simulink model "AirDirecCtrlMgr"  (R2022b)
settings: Solver=FixedStepDiscrete  StartTime=0.0  StopTime=10.0  FixedStep=0.2  RelTol=1e-3
(part 1 of 2)
settings Model: Creator=blue.ge  LastModifiedBy=blue.ge
settings DataIOCC: SaveFormat=StructureWithTime
settings OptimizationCC: DefaultParameterBehavior=Inlined  EnhancedBackFolding=on  PassReuseOutputArgsAs=Individual arguments  ZeroExternalMemoryAtStartup=off  ZeroInternalMemoryAtStartup=off  OptimizeBlockOrder=speed  DifferentSizesBufferReuse=on  OptimizationCustomize=off  EfficientTunableParamExpr=on
settings DebuggingCC: ParameterTunabilityLossMsg=error
settings ModelReferenceCC: ModelReferenceNumInstancesAllowed=Single
settings SFSimCC: MATLABDynamicMemAlloc=off
settings RTWCC: Description=AUTOSAR  SystemTargetFile=autosar.tlc  GenCodeOnly=on  TemplateMakefile=ert_default_tmf
settings CodeAppCC: ForceParamTrailComments=on  IgnoreCustomStorageClasses=off  OperatorAnnotations=on  SimulinkDataObjDesc=on  SFDataObjDesc=on  InsertBlockDesc=on
settings STFCustomTargetCC: TargetLangStandard=C99 (ISO)  MultiwordLength=256  ParMdlRefBuildCompliant=on  CompOptLevelCompliant=on  IncludeMdlTerminateFcn=off  CombineOutputUpdateFcns=on  SuppressErrorStatus=on  ERTCustomFileBanners=on  SupportNonFinite=off  SupportComplex=off  SupportContinuousTime=off  SupportNonInlinedSFcns=off  RemoveResetFunc=on  MATLABClassNameForMDSCustomization=Simulink.SoftwareTarget.ERTCustomization  AutosarCompliant=on  UseToolchainInfoCompliant=on  LookupTableObjectStructAxisOrder=2,1,3,4,...  LUTObjectStructOrderEvenSpacing=Size,Table,Breakpoints  SystemTargetFile=autosar.tlc
settings ERTTargetCC: TargetLangStandard=C99 (ISO)  ConcurrentExecutionCompliant=off  IncludeMdlTerminateFcn=off  SuppressErrorStatus=on  SupportNonFinite=off  SupportComplex=off  ModelStepFunctionPrototypeControlCompliant=off  CPPClassGenCompliant=off  AutosarCompliant=on  LookupTableObjectStructAxisOrder=2,1,3,4,...  LUTObjectStructOrderEvenSpacing=Size,Table,Breakpoints  InlinedParameterPlacement=NonHierarchical  ERTCustomFileTemplate=example_file_process.tlc

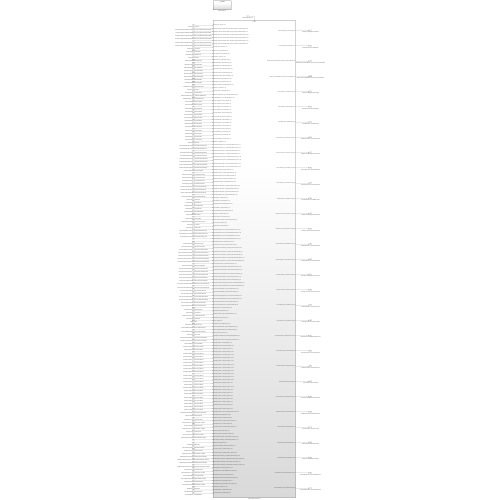
[thumbnail: Simulink's stored preview of the top-level diagram]
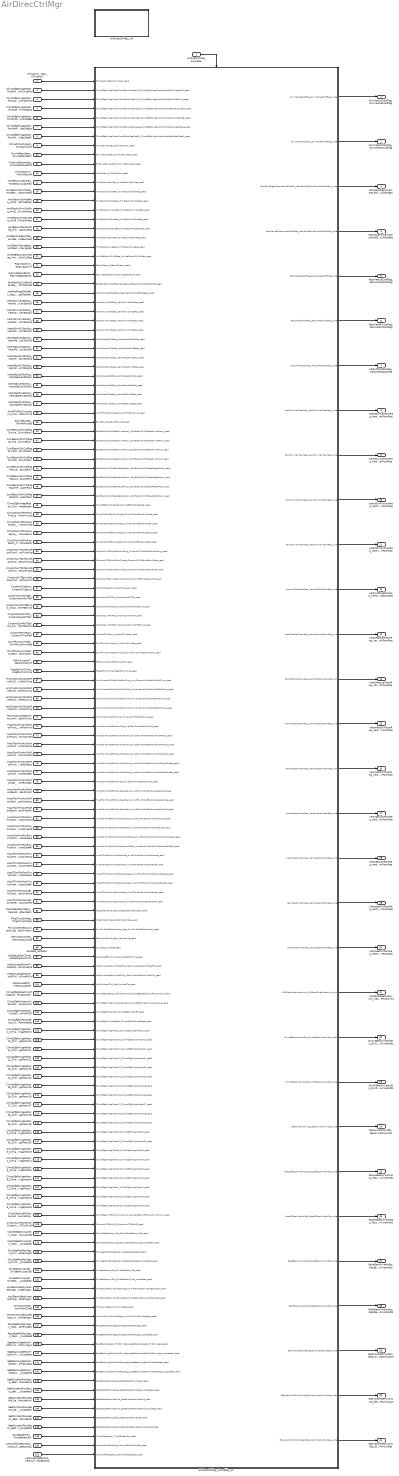
== system AirDirecCtrlMgr ==
  AirDirecCtrlMgr_Runnable : Inport  SampleTime=0.2  OutputFunctionCall=on
  ClimaActv_Ngcc_ClimaActv : Inport  Port=2  OutDataTypeStr=Enum: OnOff1  PortDimensions=1
  ClimaCfgForVgaMotorPrsntAtRowFirstLeMid_ClimaCfgForVgaMotorPrsntAtRowFirstLeMid : Inport  Port=3  OutDataTypeStr=Boolean  PortDimensions=1
  ClimaCfgForVgaMotorPrsntAtRowFirstRiMid_ClimaCfgForVgaMotorPrsntAtRowFirstRiMid : Inport  Port=4  OutDataTypeStr=Boolean  PortDimensions=1
  ClimaCfgForVgaMotorPrsntAtRowFirstSideLe_ClimaCfgForVgaMotorPrsntAtRowFirstSideLe : Inport  Port=5  OutDataTypeStr=Boolean  PortDimensions=1
  ClimaCfgForVgaMotorPrsntAtRowFirstSideRi_ClimaCfgForVgaMotorPrsntAtRowFirstSideRi : Inport  Port=6  OutDataTypeStr=Boolean  PortDimensions=1
  ClimaCfgForVgaMotorPrsntAtRowSecSideLe_ClimaCfgForVgaMotorPrsntAtRowSecSideLe : Inport  Port=7  OutDataTypeStr=Boolean  PortDimensions=1
  ClimaCfgForVgaMotorPrsntAtRowSecSideRi_ClimaCfgForVgaMotorPrsntAtRowSecSideRi : Inport  Port=8  OutDataTypeStr=Boolean  PortDimensions=1
  ClimaCtrlActvNgcc_ClimaCtrlActv : Inport  Port=9  OutDataTypeStr=Boolean  PortDimensions=1
  DrvrHdPosnDetn_DrvrHdPosnDetn : Inport  Port=10  OutDataTypeStr=Bus: Detn20  PortDimensions=1  BusOutputAsStruct=on
  FrntHvacBlowerSts_FrntHvacBlowerSts : Inport  Port=11  OutDataTypeStr=Enum: HvacFanSts  PortDimensions=1
  FrntVGAerror_FrntVGAerror : Inport  Port=12  OutDataTypeStr=Boolean  PortDimensions=1
  HmiElecAirAdpvReq_HmiElecAirAdpvReq : Inport  Port=13  OutDataTypeStr=Bus: AdpvReq  PortDimensions=1  BusOutputAsStruct=on
  HmiElecAirDirCrtlReq_HmiElecAirDirCrtlReq : Inport  Port=14  OutDataTypeStr=Bus: HmiElecAirDirCrtlReq  PortDimensions=1  BusOutputAsStruct=on
  HmiElecAirDirModReq_HmiElecAirDirModReq : Inport  Port=15  OutDataTypeStr=Bus: HmiElecAirDirModReq  PortDimensions=1  BusOutputAsStruct=on
  HmiElecAirDrvrSwtReq_HmiElecAirDrvrSwtReq : Inport  Port=16  OutDataTypeStr=Enum: OnOff1  PortDimensions=1
  HmiElecAirFrntSwtReq_HmiElecAirFrntSwtReq : Inport  Port=17  OutDataTypeStr=Bus: SwtReq3  PortDimensions=1  BusOutputAsStruct=on
  HmiElecAirPassSwtReq_HmiElecAirPassSwtReq : Inport  Port=18  OutDataTypeStr=Enum: OnOff1  PortDimensions=1
  HmiElecAirReSwtReq_HmiElecAirReSwtReq : Inport  Port=19  OutDataTypeStr=Bus: SwtReq4  PortDimensions=1  BusOutputAsStruct=on
  HmiElecAirSwngReq_HmiElecAirSwngReq : Inport  Port=20  OutDataTypeStr=Bus: HmiElecAirSwngReq  PortDimensions=1  BusOutputAsStruct=on
  HmiReElecAirDirCrtlReq_HmiReElecAirDirCrtlReq : Inport  Port=21  OutDataTypeStr=Bus: PosIndct  PortDimensions=1  BusOutputAsStruct=on
  RearVGAerror_RearVGAerror : Inport  Port=22  OutDataTypeStr=Boolean  PortDimensions=1
  ResrvdSigForECM4_ResrvdSigForECM4 : Inport  Port=23  OutDataTypeStr=BattSnsrChksTbl  PortDimensions=1
  StrtRemSts1WdStrtRemSts_Ngcc_StrtRemSts1WdStrtRemSts : Inport  Port=24  OutDataTypeStr=Enum: StrtRemSts1  PortDimensions=1
  VehModMngtGlbSafe1_Ngcc_VehModMngtGlbSafe1 : Inport  Port=25  OutDataTypeStr=Bus: VehModMngtGlbSafe1  PortDimensions=1  BusOutputAsStruct=on
  VentnDrvrLeXStsRsp_VentnDrvrLeXStsRsp : Inport  Port=26  OutDataTypeStr=Enum: VentnStsRsp  PortDimensions=1
  VentnDrvrLeYStsRsp_VentnDrvrLeYStsRsp : Inport  Port=27  OutDataTypeStr=Enum: VentnStsRsp  PortDimensions=1
  VentnDrvrRiXStsRsp_VentnDrvrRiXStsRsp : Inport  Port=28  OutDataTypeStr=Enum: VentnStsRsp  PortDimensions=1
  VentnDrvrRiYStsRsp_VentnDrvrRiYStsRsp : Inport  Port=29  OutDataTypeStr=Enum: VentnStsRsp  PortDimensions=1
  VentnPassLeXStsRsp_VentnPassLeXStsRsp : Inport  Port=30  OutDataTypeStr=Enum: VentnStsRsp  PortDimensions=1
  VentnPassLeYStsRsp_VentnPassLeYStsRsp : Inport  Port=31  OutDataTypeStr=Enum: VentnStsRsp  PortDimensions=1
  VentnPassRiXStsRsp_VentnPassRiXStsRsp : Inport  Port=32  OutDataTypeStr=Enum: VentnStsRsp  PortDimensions=1
  VentnPassRiYStsRsp_VentnPassRiYStsRsp : Inport  Port=33  OutDataTypeStr=Enum: VentnStsRsp  PortDimensions=1
  VentnSecLeXStsRsp_VentnSecLeXStsRsp : Inport  Port=34  OutDataTypeStr=Enum: VentnStsRsp  PortDimensions=1
  VentnSecLeYStsRsp_VentnSecLeYStsRsp : Inport  Port=35  OutDataTypeStr=Enum: VentnStsRsp  PortDimensions=1
  VentnSecRiXStsRsp_VentnSecRiXStsRsp : Inport  Port=36  OutDataTypeStr=Enum: VentnStsRsp  PortDimensions=1
  VentnSecRiYStsRsp_VentnSecRiYStsRsp : Inport  Port=37  OutDataTypeStr=Enum: VentnStsRsp  PortDimensions=1
  AmbTFildForClimaNgcc_AmbTFildForClima : Inport  Port=38  OutDataTypeStr=T30  PortDimensions=1
  BlwAftRunSts_BlwAftRunSts : Inport  Port=39  OutDataTypeStr=Boolean  PortDimensions=1
  CcmElecAirDirCrtlReqDrvrLePosX_CcmElecAirDirCrtlReqDrvrLePosX : Inport  Port=40  OutDataTypeStr=UInt8  PortDimensions=1
  CcmElecAirDirCrtlReqDrvrLePosY_CcmElecAirDirCrtlReqDrvrLePosY : Inport  Port=41  OutDataTypeStr=UInt8  PortDimensions=1
  CcmElecAirDirCrtlReqDrvrRiPosX_CcmElecAirDirCrtlReqDrvrRiPosX : Inport  Port=42  OutDataTypeStr=UInt8  PortDimensions=1
  CcmElecAirDirCrtlReqDrvrRiPosY_CcmElecAirDirCrtlReqDrvrRiPosY : Inport  Port=43  OutDataTypeStr=UInt8  PortDimensions=1
  CcmElecAirDirCrtlReqPassLePosX_CcmElecAirDirCrtlReqPassLePosX : Inport  Port=44  OutDataTypeStr=UInt8  PortDimensions=1
  CcmElecAirDirCrtlReqPassLePosY_CcmElecAirDirCrtlReqPassLePosY : Inport  Port=45  OutDataTypeStr=UInt8  PortDimensions=1
  CcmElecAirDirCrtlReqPassRiPosX_CcmElecAirDirCrtlReqPassRiPosX : Inport  Port=46  OutDataTypeStr=UInt8  PortDimensions=1
  CcmElecAirDirCrtlReqPassRiPosY_CcmElecAirDirCrtlReqPassRiPosY : Inport  Port=47  OutDataTypeStr=UInt8  PortDimensions=1
  ClimaCfgForHeadRadar_ClimaCfgForHeadRadar : Inport  Port=48  OutDataTypeStr=Enum: HeadRadar  PortDimensions=1
  ClimaTAtCmftForRowFirstLe_ClimaTAtCmftForRowFirstLe : Inport  Port=49  OutDataTypeStr=T30  PortDimensions=1
  ClimaTAtCmftForRowFirstRi_ClimaTAtCmftForRowFirstRi : Inport  Port=50  OutDataTypeStr=T30  PortDimensions=1
  ClimaTAtCmftForRowSecLe_ClimaTAtCmftForRowSecLe : Inport  Port=51  OutDataTypeStr=T30  PortDimensions=1
  ClimaTAtCmftForRowSecRi_ClimaTAtCmftForRowSecRi : Inport  Port=52  OutDataTypeStr=T30  PortDimensions=1
  CmptmtAirTCtrlErrAtRowFirstAvg_CmptmtAirTCtrlErrAtRowFirstAvg : Inport  Port=53  OutDataTypeStr=T30  PortDimensions=1
  CmptmtAirTCtrlErrAtRowFirstLe_CmptmtAirTCtrlErrAtRowFirstLe : Inport  Port=54  OutDataTypeStr=T30  PortDimensions=1
  CmptmtAirTCtrlErrAtRowFirstRi_CmptmtAirTCtrlErrAtRowFirstRi : Inport  Port=55  OutDataTypeStr=T30  PortDimensions=1
  CmptmtAirTEstimdAtRowFirst_CmptmtAirTEstimdAtRowFirst : Inport  Port=56  OutDataTypeStr=T30  PortDimensions=1
  CmptmtClngActv_CmptmtClngActv : Inport  Port=57  OutDataTypeStr=Enum: CmptmtClngActv  PortDimensions=1
  CmptmtIntrMtrlTErr_CmptmtIntrMtrlTErr : Inport  Port=58  OutDataTypeStr=T30  PortDimensions=1
  CmptmtIntrMtrlTEstimd_CmptmtIntrMtrlTEstimd : Inport  Port=59  OutDataTypeStr=Bus: MtrlSnsrT  PortDimensions=1  BusOutputAsStruct=on
  CmptmtSunMtrlTErr_CmptmtSunMtrlTErr : Inport  Port=60  OutDataTypeStr=T30  PortDimensions=1
  CmptmtSunMtrlTEstimd_CmptmtSunMtrlTEstimd : Inport  Port=61  OutDataTypeStr=T30  PortDimensions=1
  CmptmtTFrntRaw_CmptmtTFrntRaw : Inport  Port=62  OutDataTypeStr=Bus: CmptmtTFrnt  PortDimensions=1  BusOutputAsStruct=on
  DrvrPfmncAlrmReq_DrvrPfmncAlrmReq : Inport  Port=63  OutDataTypeStr=Enum: DrvrPfmncWarnReq  PortDimensions=1
  DrvrPfmncAlrmReqFromDHU_DrvrPfmncAlrmReqFromDHU : Inport  Port=64  OutDataTypeStr=Enum: DrvrPfmncWarnReq  PortDimensions=1
  FbForCmptmtT_FbForCmptmtT : Inport  Port=65  OutDataTypeStr=T30  PortDimensions=1
  FragStsFrmClima_FragStsFrmClima : Inport  Port=66  OutDataTypeStr=Enum: AirFragSts  PortDimensions=1
  HmiCmptmtAirDistbnFrntForClima_HmiCmptmtAirDistbnFrntForClima : Inport  Port=67  OutDataTypeStr=Enum: HmiCmptmtAirDistbnFrnt  PortDimensions=1
  HmiCmptmtAirDistbnFrntForClima_HmiCmptmtAirDistbnFrntRiForClima : Inport  Port=68  OutDataTypeStr=Enum: HmiCmptmtAirDistbnFrnt  PortDimensions=1
  HmiCmptmtAirDistbnFrntForClima_HmiCmptmtAirDistbnReForClima : Inport  Port=69  OutDataTypeStr=Enum: HmiCmptmtAirDistbnFrnt  PortDimensions=1
  HmiCmptmtAirDistbnFrntForClima_HmiCmptmtAirDistbnReRiForClima : Inport  Port=70  OutDataTypeStr=Enum: HmiCmptmtAirDistbnFrnt  PortDimensions=1
  HmiCmptmtTSpForClima_HmiCmptmtTSpForClima : Inport  Port=71  OutDataTypeStr=Bus: HmiCmptmtTSp  PortDimensions=1  BusOutputAsStruct=on
  HvacFlowFrntActForRowFirst_HvacFlowFrntActForRowFirst : Inport  Port=72  OutDataTypeStr=AirFlow30  PortDimensions=1
  HvacFlowFrntActForRowFirstAtVent_HvacFlowFrntActForRowFirstAtVent : Inport  Port=73  OutDataTypeStr=AirFlow30  PortDimensions=1
  HvacFlowFrntActForRowFirstAtVent_HvacFlowFrntActForRowFirstAtVentLe : Inport  Port=74  OutDataTypeStr=AirFlow30  PortDimensions=1
  HvacFlowFrntActForRowFirstAtVent_HvacFlowFrntActForRowFirstAtVentRi : Inport  Port=75  OutDataTypeStr=AirFlow30  PortDimensions=1
  HvacFlowFrntActForRowFirstAtVent_HvacFlowFrntActForRowFirstAtVentSideLe : Inport  Port=76  OutDataTypeStr=AirFlow30  PortDimensions=1
  HvacFlowFrntActForRowFirstAtVent_HvacFlowFrntActForRowFirstAtVentSideRi : Inport  Port=77  OutDataTypeStr=AirFlow30  PortDimensions=1
  HvacFlowFrntActForRowSec_HvacFlowFrntActForRowSec : Inport  Port=78  OutDataTypeStr=AirFlow30  PortDimensions=1
  HvacFlowFrntActForRowSecAtVent_HvacFlowFrntActForRowSecAtVent : Inport  Port=79  OutDataTypeStr=AirFlow30  PortDimensions=1
  HvacFlowFrntActForRowSecAtVent_HvacFlowFrntActForRowSecAtVentLe : Inport  Port=80  OutDataTypeStr=AirFlow30  PortDimensions=1
  HvacFlowFrntActForRowSecAtVent_HvacFlowFrntActForRowSecAtVentRi : Inport  Port=81  OutDataTypeStr=AirFlow30  PortDimensions=1
  HvacFlowFrntForRowFirstAtVentLe_HvacFlowFrntForRowFirstAtVentLe : Inport  Port=82  OutDataTypeStr=AirFlow30  PortDimensions=1
  HvacFlowFrntForRowFirstAtVentRi_HvacFlowFrntForRowFirstAtVentRi : Inport  Port=83  OutDataTypeStr=AirFlow30  PortDimensions=1
  HvacFlowFrntForRowFirstAtVentSideLe_HvacFlowFrntForRowFirstAtVentSideLe : Inport  Port=84  OutDataTypeStr=AirFlow30  PortDimensions=1
  HvacFlowFrntForRowFirstAtVentSideRi_HvacFlowFrntForRowFirstAtVentSideRi : Inport  Port=85  OutDataTypeStr=AirFlow30  PortDimensions=1
  HvacTFrntForRowFirstAtVentLe_HvacTFrntForRowFirstAtVentLe : Inport  Port=86  OutDataTypeStr=T30  PortDimensions=1
  HvacTFrntForRowFirstAtVentRi_HvacTFrntForRowFirstAtVentRi : Inport  Port=87  OutDataTypeStr=T30  PortDimensions=1
  HvacTFrntForRowFirstAtVentSideLe_HvacTFrntForRowFirstAtVentSideLe : Inport  Port=88  OutDataTypeStr=T30  PortDimensions=1
  HvacTFrntForRowFirstAtVentSideRi_HvacTFrntForRowFirstAtVentSideRi : Inport  Port=89  OutDataTypeStr=T30  PortDimensions=1
  HvacTFrntForRowSecAtVentLe_HvacTFrntForRowSecAtVentLe : Inport  Port=90  OutDataTypeStr=T30  PortDimensions=1
  HvacTFrntForRowSecAtVentRi_HvacTFrntForRowSecAtVentRi : Inport  Port=91  OutDataTypeStr=T30  PortDimensions=1
  PassSideHdPosnDetn_PassSideHdPosnDetn : Inport  Port=92  OutDataTypeStr=Bus: Detn20  PortDimensions=1  BusOutputAsStruct=on
  PrkgClimaOnReq_PrkgClimaOnReq : Inport  Port=93  OutDataTypeStr=Boolean  PortDimensions=1
  PwrAllwdForElecAirVentn_Ngcc_PwrAllwdForElecAirVentn : Inport  Port=94  OutDataTypeStr=ScrHeatrEgyPwrCns1  PortDimensions=1
  RemVentActvSts_RemVentActvSts : Inport  Port=95  OutDataTypeStr=Enum: OnOff1  PortDimensions=1
  SunData_SunData : Inport  Port=96  OutDataTypeStr=Bus: SunData  PortDimensions=1  BusOutputAsStruct=on
  VehSpdLgtForClima_VehSpdLgtForClima : Inport  Port=97  OutDataTypeStr=Bus: VehSpdLgtForClima  PortDimensions=1  BusOutputAsStruct=on
  VehSunLoadAtRowFirstLeFild_VehSunLoadAtRowFirstLeFild : Inport  Port=98  OutDataTypeStr=SunLoad30  PortDimensions=1
  VehSunLoadAtRowFirstRiFild_VehSunLoadAtRowFirstRiFild : Inport  Port=99  OutDataTypeStr=SunLoad30  PortDimensions=1
  VehSunLoadTot_VehSunLoadTot : Inport  Port=100  OutDataTypeStr=SunLoad30  PortDimensions=1
  ClimaCfgForElectricLiftingModule_ClimaCfgForElectricLiftingModule : Inport  Port=101  OutDataTypeStr=Enum: ElcTrLFtngMdle  PortDimensions=1
  ClimaCfgForInteriorViewCamera_ClimaCfgForInteriorViewCamera : Inport  Port=102  OutDataTypeStr=Enum: CarCfgForInteriorViewCamera  PortDimensions=1
  ClimaCfgForMARKET_ClimaCfgForMARKET : Inport  Port=103  OutDataTypeStr=UInt8  PortDimensions=1
  ClimaCfgForPowerSeats_ClimaCfgForPowerSeats : Inport  Port=104  OutDataTypeStr=Enum: CarCfgForPowerSeats  PortDimensions=1
  ClimaCfgForVgaMotor1_ClimaCfgForVgaMotor1 : Inport  Port=105  OutDataTypeStr=Boolean  PortDimensions=1
  ClimaCfgForVgaMotor10_ClimaCfgForVgaMotor10 : Inport  Port=106  OutDataTypeStr=Boolean  PortDimensions=1
  ClimaCfgForVgaMotor11_ClimaCfgForVgaMotor11 : Inport  Port=107  OutDataTypeStr=Boolean  PortDimensions=1
  ClimaCfgForVgaMotor12_ClimaCfgForVgaMotor12 : Inport  Port=108  OutDataTypeStr=Boolean  PortDimensions=1
  ClimaCfgForVgaMotor13_ClimaCfgForVgaMotor13 : Inport  Port=109  OutDataTypeStr=Boolean  PortDimensions=1
  ClimaCfgForVgaMotor14_ClimaCfgForVgaMotor14 : Inport  Port=110  OutDataTypeStr=Boolean  PortDimensions=1
  ClimaCfgForVgaMotor15_ClimaCfgForVgaMotor15 : Inport  Port=111  OutDataTypeStr=Boolean  PortDimensions=1
  ClimaCfgForVgaMotor16_ClimaCfgForVgaMotor16 : Inport  Port=112  OutDataTypeStr=Boolean  PortDimensions=1
  ClimaCfgForVgaMotor17_ClimaCfgForVgaMotor17 : Inport  Port=113  OutDataTypeStr=Boolean  PortDimensions=1
  ClimaCfgForVgaMotor18_ClimaCfgForVgaMotor18 : Inport  Port=114  OutDataTypeStr=Boolean  PortDimensions=1
  ClimaCfgForVgaMotor19_ClimaCfgForVgaMotor19 : Inport  Port=115  OutDataTypeStr=Boolean  PortDimensions=1
  ClimaCfgForVgaMotor2_ClimaCfgForVgaMotor2 : Inport  Port=116  OutDataTypeStr=Boolean  PortDimensions=1
  ClimaCfgForVgaMotor20_ClimaCfgForVgaMotor20 : Inport  Port=117  OutDataTypeStr=Boolean  PortDimensions=1
  ClimaCfgForVgaMotor3_ClimaCfgForVgaMotor3 : Inport  Port=118  OutDataTypeStr=Boolean  PortDimensions=1
  ClimaCfgForVgaMotor4_ClimaCfgForVgaMotor4 : Inport  Port=119  OutDataTypeStr=Boolean  PortDimensions=1
  ClimaCfgForVgaMotor5_ClimaCfgForVgaMotor5 : Inport  Port=120  OutDataTypeStr=Boolean  PortDimensions=1
  ClimaCfgForVgaMotor6_ClimaCfgForVgaMotor6 : Inport  Port=121  OutDataTypeStr=Boolean  PortDimensions=1
  ClimaCfgForVgaMotor7_ClimaCfgForVgaMotor7 : Inport  Port=122  OutDataTypeStr=Boolean  PortDimensions=1
  ClimaCfgForVgaMotor8_ClimaCfgForVgaMotor8 : Inport  Port=123  OutDataTypeStr=Boolean  PortDimensions=1
  ClimaCfgForVgaMotor9_ClimaCfgForVgaMotor9 : Inport  Port=124  OutDataTypeStr=Boolean  PortDimensions=1
  ClimaCfgWindFollowHumanMov_ClimaCfgWindFollowHumanMov : Inport  Port=125  OutDataTypeStr=Enum: WndFllwngHmnMvmntFnctn  PortDimensions=1
  CmptmtAirTCtrlErrQf_CmptmtAirTCtrlErrQf : Inport  Port=126  OutDataTypeStr=Enum: HvacTIfQf  PortDimensions=1
  CopilotSeatOccupySts_CopilotSeatOccupySts : Inport  Port=127  OutDataTypeStr=Enum: ActvnAvl3  PortDimensions=1
  CopilotSeatOccupySts_CopilotSeatOccupySts_IsUpdated : Inport  Port=128  OutDataTypeStr=boolean  PortDimensions=1
  DrvrSeatPosPercNgcc_DrvrSeatPosPercNgcc : Inport  Port=129  OutDataTypeStr=Bus: SeatPosPercQF  PortDimensions=1  BusOutputAsStruct=on
  DrvrSeatPosPercNgcc_DrvrSeatPosPercNgcc_IsUpdated : Inport  Port=130  OutDataTypeStr=boolean  PortDimensions=1
  DrvSeatOccupySts_DrvSeatOccupySts : Inport  Port=131  OutDataTypeStr=Enum: ActvnAvl3  PortDimensions=1
  DrvSeatOccupySts_DrvSeatOccupySts_IsUpdated : Inport  Port=132  OutDataTypeStr=boolean  PortDimensions=1
  HmiElecAirBodyVentLebtnNgcc_HmiElecAirBodyVentLebtnNgcc : Inport  Port=133  OutMin=0  OutMax=15  OutDataTypeStr=UInt4  PortDimensions=1
  HmiElecAirBodyVentRibtnNgcc_HmiElecAirBodyVentRibtnNgcc : Inport  Port=134  OutMin=0  OutMax=15  OutDataTypeStr=UInt4  PortDimensions=1
  HmiMaxACReq_HmiMaxACReq : Inport  Port=135  OutDataTypeStr=Enum: OnOff1  PortDimensions=1
  HmiSmrtWindFolwSetNgcc_HmiSmrtWindFolwSetNgcc : Inport  Port=136  OutDataTypeStr=Enum: OnOff1  PortDimensions=1
  PassSeatPosPercNgcc_PassSeatPosPercNgcc : Inport  Port=137  OutDataTypeStr=Bus: SeatPosPercQF  PortDimensions=1  BusOutputAsStruct=on
  PassSeatPosPercNgcc_PassSeatPosPercNgcc_IsUpdated : Inport  Port=138  OutDataTypeStr=boolean  PortDimensions=1
  SeatBackAngleRowFirstDrvrNgcc_SeatBackAngleRowFirstDrvrNgcc : Inport  Port=139  OutMin=0  OutMax=180  OutDataTypeStr=AngleDeg180  PortDimensions=1
  SeatBackAngleRowFirstDrvrNgcc_SeatBackAngleRowFirstDrvrNgcc_IsUpdated : Inport  Port=140  OutDataTypeStr=boolean  PortDimensions=1
  SeatBackAngleRowFirstPassNgcc_SeatBackAngleRowFirstPassNgcc : Inport  Port=141  OutMin=0  OutMax=180  OutDataTypeStr=AngleDeg180  PortDimensions=1
  SeatBackAngleRowFirstPassNgcc_SeatBackAngleRowFirstPassNgcc_IsUpdated : Inport  Port=142  OutDataTypeStr=boolean  PortDimensions=1
  SeatOccptAtRowSecLe_SeatOccptAtRowSecLe : Inport  Port=143  OutDataTypeStr=Enum: PassSeatSts1  PortDimensions=1
  SeatOccptAtRowSecLe_SeatOccptAtRowSecLe_IsUpdated : Inport  Port=144  OutDataTypeStr=boolean  PortDimensions=1
  SeatOccptAtRowSecMid_SeatOccptAtRowSecMid : Inport  Port=145  OutDataTypeStr=Enum: PassSeatSts1  PortDimensions=1
  SeatOccptAtRowSecMid_SeatOccptAtRowSecMid_IsUpdated : Inport  Port=146  OutDataTypeStr=boolean  PortDimensions=1
  SeatOccptAtRowSecRi_SeatOccptAtRowSecRi : Inport  Port=147  OutDataTypeStr=Enum: PassSeatSts1  PortDimensions=1
  SeatOccptAtRowSecRi_SeatOccptAtRowSecRi_IsUpdated : Inport  Port=148  OutDataTypeStr=boolean  PortDimensions=1
  TiAndDateIndcn_TiAndDateIndcn : Inport  Port=149  OutDataTypeStr=Bus: DateTi30  PortDimensions=1  BusOutputAsStruct=on
  VentnLiftModPosnRsp_VentnLiftModPosnRsp : Inport  Port=150  OutDataTypeStr=UInt8  PortDimensions=1
  VentnLiftModStsRsp_VentnLiftModStsRsp : Inport  Port=151  OutDataTypeStr=Enum: VentnStsRsp  PortDimensions=1
  AirDirecCtrlMgr_Init : Subsystem
  AirDirecCtrlMgr_Runnable_sys : Subsystem
  DrvrVentLeClsdFlag_DrvrVentLeClsdFlag : Outport  OutDataTypeStr=Boolean  PortDimensions=1
  DrvrVentRiClsdFlag_DrvrVentRiClsdFlag : Outport  Port=2  OutDataTypeStr=Boolean  PortDimensions=1
  MemSaveFlgForCenVentLiftModErr_MemSaveFlgForCenVentLiftModErr : Outport  Port=3  OutDataTypeStr=Boolean  PortDimensions=1
  MemSaveForCenVentLiftModSts_MemSaveForCenVentLiftModSts : Outport  Port=4  OutDataTypeStr=Bus: MemSaveLiftMod  PortDimensions=1  BusOutputAsStruct=on
  PassVentLeClsdFlag_PassVentLeClsdFlag : Outport  Port=5  OutDataTypeStr=Boolean  PortDimensions=1
  PassVentRiClsdFlag_PassVentRiClsdFlag : Outport  Port=6  OutDataTypeStr=Boolean  PortDimensions=1
  VentLiftModCaliReq_VentLiftModCaliReq : Outport  Port=7  OutDataTypeStr=UInt8  PortDimensions=1
  VentnDrvrLeXPsonReq_VentnDrvrLeXPsonReq : Outport  Port=8  OutDataTypeStr=UInt16  PortDimensions=1
  VentnDrvrLeYPsonReq_VentnDrvrLeYPsonReq : Outport  Port=9  OutDataTypeStr=UInt16  PortDimensions=1
  VentnDrvrRiXPsonReq_VentnDrvrRiXPsonReq : Outport  Port=10  OutDataTypeStr=UInt16  PortDimensions=1
  VentnDrvrRiYPsonReq_VentnDrvrRiYPsonReq : Outport  Port=11  OutDataTypeStr=UInt16  PortDimensions=1
  VentnLiftModPosnReq_VentnLiftModPosnReq : Outport  Port=12  OutDataTypeStr=UInt8  PortDimensions=1
  VentnPassLeXPsonReq_VentnPassLeXPsonReq : Outport  Port=13  OutDataTypeStr=UInt16  PortDimensions=1
  VentnPassLeYPsonReq_VentnPassLeYPsonReq : Outport  Port=14  OutDataTypeStr=UInt16  PortDimensions=1
  VentnPassRiXPsonReq_VentnPassRiXPsonReq : Outport  Port=15  OutDataTypeStr=UInt16  PortDimensions=1
  VentnPassRiYPsonReq_VentnPassRiYPsonReq : Outport  Port=16  OutDataTypeStr=UInt16  PortDimensions=1
  VentnSecLeXPosnReq_VentnSecLeXPosnReq : Outport  Port=17  OutDataTypeStr=UInt16  PortDimensions=1
  VentnSecLeYPosnReq_VentnSecLeYPosnReq : Outport  Port=18  OutDataTypeStr=UInt16  PortDimensions=1
  VentnSecRiXPosnReq_VentnSecRiXPosnReq : Outport  Port=19  OutDataTypeStr=UInt16  PortDimensions=1
  VentnSecRiYPosnReq_VentnSecRiYPosnReq : Outport  Port=20  OutDataTypeStr=UInt16  PortDimensions=1
  VGAStopDuePowerLimit_VGAStopDuePowerLimit : Outport  Port=21  OutDataTypeStr=Boolean  PortDimensions=1
  DrvrLeElecAirVentnSts_DrvrLeElecAirVentnSts : Outport  Port=22  OutDataTypeStr=Enum: OnOff1  PortDimensions=1
  DrvrRiElecAirVentnSts_DrvrRiElecAirVentnSts : Outport  Port=23  OutDataTypeStr=Enum: OnOff1  PortDimensions=1
  ElecAirVentnAvlSts_ElecAirVentnAvlSts : Outport  Port=24  OutDataTypeStr=Enum: StsFd  PortDimensions=1
  PassLeElecAirVentnSts_PassLeElecAirVentnSts : Outport  Port=25  OutDataTypeStr=Enum: OnOff1  PortDimensions=1
  PassRiElecAirVentnSts_PassRiElecAirVentnSts : Outport  Port=26  OutDataTypeStr=Enum: OnOff1  PortDimensions=1
  ReLeElecAirVentnSts_ReLeElecAirVentnSts : Outport  Port=27  OutDataTypeStr=Enum: OnOff1  PortDimensions=1
  ReRiElecAirVentnSts_ReRiElecAirVentnSts : Outport  Port=28  OutDataTypeStr=Enum: OnOff1  PortDimensions=1
  EleAirOutletCmpFact_EleAirOutletCmpFact : Outport  Port=29  OutDataTypeStr=VCCFac30  PortDimensions=1
  ElecVentnMotPwrCnsAct_ElecVentnMotPwrCnsAct : Outport  Port=30  OutDataTypeStr=ScrHeatrEgyPwrCns1  PortDimensions=1
  ElecVentnMotPwrCnsDes_ElecVentnMotPwrCnsDes : Outport  Port=31  OutDataTypeStr=ScrHeatrEgyPwrCns1  PortDimensions=1
  wires:
  ClimaActv_Ngcc_ClimaActv/1 -> AirDirecCtrlMgr_Runnable_sys/1(ClimaActv_Ngcc_ClimaActv_read)
  ClimaCfgForVgaMotorPrsntAtRowFirstLeMid_ClimaCfgForVgaMotorPrsntAtRowFirstLeMid/1 -> AirDirecCtrlMgr_Runnable_sys/2(ClimaCfgForVgaMotorPrsntAtRowFirstLeMid_ClimaCfgForVgaMotorPrsntAtRowFirstLeMid_read)
  ClimaCfgForVgaMotorPrsntAtRowFirstRiMid_ClimaCfgForVgaMotorPrsntAtRowFirstRiMid/1 -> AirDirecCtrlMgr_Runnable_sys/3(ClimaCfgForVgaMotorPrsntAtRowFirstRiMid_ClimaCfgForVgaMotorPrsntAtRowFirstRiMid_read)